simulink model "MX_Gateway"  (Simulink 9.0)
settings: Solver=FixedStepDiscrete  StartTime=0.0  StopTime=100  FixedStep=0.01  RelTol=1e-3
values from neighbouring files (symbols this model uses):
  ACTIVE = Bobcat.Parameter; CreateDefine( ACTIVE                         ,1                          ,'Define' ,'defines.h' )    [defines.m]
  ACTIVE_MODE_ONE = Bobcat.Parameter; CreateDefine( ACTIVE_MODE_ONE                ,19                         ,'Define' ,'defines.h' )    [defines.m]
  AUTHENTICATED = Bobcat.Parameter; CreateDefine( AUTHENTICATED                  ,1                          ,'Define' ,'defines.h' )    [defines.m]
  AUX_EMEA_CONTROLS = Bobcat.Parameter; CreateDefine( AUX_EMEA_CONTROLS  ,1   ,'Define' ,'defines.h' )    [enums.m]
  AUX_FLOW_HIGH = Bobcat.Parameter; CreateDefine( AUX_FLOW_HIGH           ,4  ,'Define' ,'defines.h' )    [enums.m]
  AUX_FLOW_INACTIVE = Bobcat.Parameter; CreateDefine( AUX_FLOW_INACTIVE       ,0  ,'Define' ,'defines.h' )    [enums.m]
  AUX_FLOW_LOW = Bobcat.Parameter; CreateDefine( AUX_FLOW_LOW            ,1  ,'Define' ,'defines.h' )    [enums.m]
  AUX_FLOW_MEDIUM = Bobcat.Parameter; CreateDefine( AUX_FLOW_MEDIUM         ,2  ,'Define' ,'defines.h' )    [enums.m]
  AUX_FLOW_STANDARD = Bobcat.Parameter; CreateDefine( AUX_FLOW_STANDARD       ,3  ,'Define' ,'defines.h' )    [enums.m]
  AUX_NON_FUNCTIONAL = Bobcat.Parameter; CreateDefine( AUX_NON_FUNCTIONAL ,255 ,'Define' ,'defines.h' )    [enums.m]
  BASE_ACTIVE = Bobcat.Parameter; CreateDefine( BASE_ACTIVE                    ,253                        ,'Define' ,'defines.h' )    [defines.m]
  BASE_DETENT = Bobcat.Parameter; CreateDefine( BASE_DETENT                    ,1                          ,'Define' ,'defines.h' )    [defines.m]
  BEEP_NONE = Bobcat.Parameter; CreateDefine( BEEP_NONE                      ,255                        ,'Define' ,'defines.h' )    [defines.m]
  CD_AIR_FILTER_DEBOUNCE_NORMAL = Bobcat.Parameter; CreateAliasCD( CD_AIR_FILTER_DEBOUNCE_NORMAL                        ,uint16(500)                ,'ConstantDataPointer' ,'CONSTANT_DATA' ,'AIR_FILTER_DEBOUNCE_NORMAL')    [cdata.m]    (also in hc12_cdata.m; m4_cdata.m)
  CD_AIR_FILTER_DEBOUNCE_NOT_CONNECTED = Bobcat.Parameter; CreateAliasCD( CD_AIR_FILTER_DEBOUNCE_NOT_CONNECTED                 ,uint16(3000)               ,'ConstantDataPointer' ,'CONSTANT_DATA' ,'AIR_FILTER_DEBOUNCE_NOT_CONNECTED')    [cdata.m]    (also in hc12_cdata.m; m4_cdata.m)
  CD_AIR_FILTER_DEBOUNCE_PLUGGED = Bobcat.Parameter; CreateAliasCD( CD_AIR_FILTER_DEBOUNCE_PLUGGED                       ,uint16(3000)               ,'ConstantDataPointer' ,'CONSTANT_DATA' ,'AIR_FILTER_DEBOUNCE_PLUGGED')    [cdata.m]    (also in hc12_cdata.m; m4_cdata.m)
  CD_AIR_FILTER_FUNCTION = Bobcat.Parameter; CreateAliasCD( CD_AIR_FILTER_FUNCTION                               ,uint8(255)                 ,'ConstantDataPointer' ,'CONSTANT_DATA' ,'AIR_FILTER_FUNCTION')    [cdata.m]    (also in hc12_cdata.m; m4_cdata.m)
  CD_AIR_FILTER_PLUGGED_WEIGHT = Bobcat.Parameter; CreateAliasCD( CD_AIR_FILTER_PLUGGED_WEIGHT                         ,uint8(2)                   ,'ConstantDataPointer' ,'CONSTANT_DATA' ,'AIR_FILTER_PLUGGED_WEIGHT')    [cdata.m]    (also in hc12_cdata.m; m4_cdata.m)
  CD_AIR_FILTER_UNPLUGGED_WEIGHT = Bobcat.Parameter; CreateAliasCD( CD_AIR_FILTER_UNPLUGGED_WEIGHT                       ,uint8(1)                   ,'ConstantDataPointer' ,'CONSTANT_DATA' ,'AIR_FILTER_UNPLUGGED_WEIGHT')    [cdata.m]    (also in hc12_cdata.m; m4_cdata.m)
  CD_AUTOIDLE_ARMBAR_DELAY = Bobcat.Parameter; CreateAliasCD( CD_AUTOIDLE_ARMBAR_DELAY                             ,uint8(2)                   ,'ConstantDataPointer' ,'CONSTANT_DATA' ,'AUTOIDLE_ARMBAR_DELAY')    [cdata.m]    (also in hc12_cdata.m; m4_cdata.m)
  CD_AUTOIDLE_FUNCTION = Bobcat.Parameter; CreateAliasCD( CD_AUTOIDLE_FUNCTION                                 ,uint8(255)                 ,'ConstantDataPointer' ,'CONSTANT_DATA' ,'AUTOIDLE_FUNCTION')    [cdata.m]    (also in hc12_cdata.m; m4_cdata.m)
  CD_AUTOIDLE_IDLE_DELAY = Bobcat.Parameter; CreateAliasCD( CD_AUTOIDLE_IDLE_DELAY                               ,uint8(40)                  ,'ConstantDataPointer' ,'CONSTANT_DATA' ,'AUTOIDLE_IDLE_DELAY')    [cdata.m]    (also in hc12_cdata.m; m4_cdata.m)
  CD_AUTOIDLE_LOW_IDLE_START = Bobcat.Parameter; CreateAliasCD( CD_AUTOIDLE_LOW_IDLE_START                           ,uint8(255)                 ,'ConstantDataPointer' ,'CONSTANT_DATA' ,'AUTOIDLE_LOW_IDLE_START')    [cdata.m]    (also in hc12_cdata.m; m4_cdata.m)
  CD_AUTOIDLE_PRESSURE_OFF = Bobcat.Parameter; CreateAliasCD( CD_AUTOIDLE_PRESSURE_OFF                             ,uint16(300)                ,'ConstantDataPointer' ,'CONSTANT_DATA' ,'AUTOIDLE_PRESSURE_OFF')    [cdata.m]    (also in hc12_cdata.m; m4_cdata.m)
  CD_AUTOIDLE_PRESSURE_ON = Bobcat.Parameter; CreateAliasCD( CD_AUTOIDLE_PRESSURE_ON                              ,uint16(270)                ,'ConstantDataPointer' ,'CONSTANT_DATA' ,'AUTOIDLE_PRESSURE_ON')    [cdata.m]    (also in hc12_cdata.m; m4_cdata.m)
  CD_AUTOSHIFT_FUNCTION = Bobcat.Parameter; CreateAliasCD( CD_AUTOSHIFT_FUNCTION                                ,uint8(255)                 ,'ConstantDataPointer' ,'CONSTANT_DATA' ,'AUTOSHIFT_FUNCTION')    [cdata.m]    (also in hc12_cdata.m; m4_cdata.m)
  CD_AUTOSHIFT_PHIGH1 = Bobcat.Parameter; CreateAliasCD( CD_AUTOSHIFT_PHIGH1                                  ,uint16(205)                ,'ConstantDataPointer' ,'CONSTANT_DATA' ,'AUTOSHIFT_PHIGH1')    [cdata.m]    (also in hc12_cdata.m; m4_cdata.m)
  CD_AUTOSHIFT_PHIGH2 = Bobcat.Parameter; CreateAliasCD( CD_AUTOSHIFT_PHIGH2                                  ,uint16(193)                ,'ConstantDataPointer' ,'CONSTANT_DATA' ,'AUTOSHIFT_PHIGH2')    [cdata.m]    (also in hc12_cdata.m; m4_cdata.m)
  CD_AUTOSHIFT_PHIGH_TEMP1 = Bobcat.Parameter; CreateAliasCD( CD_AUTOSHIFT_PHIGH_TEMP1                             ,int16(70)                  ,'ConstantDataPointer' ,'CONSTANT_DATA' ,'AUTOSHIFT_PHIGH_TEMP1')    [cdata.m]    (also in hc12_cdata.m; m4_cdata.m)
  CD_AUTOSHIFT_PHIGH_TEMP2 = Bobcat.Parameter; CreateAliasCD( CD_AUTOSHIFT_PHIGH_TEMP2                             ,int16(160)                 ,'ConstantDataPointer' ,'CONSTANT_DATA' ,'AUTOSHIFT_PHIGH_TEMP2')    [cdata.m]    (also in hc12_cdata.m; m4_cdata.m)
  CD_AUTOSHIFT_PLOW1 = Bobcat.Parameter; CreateAliasCD( CD_AUTOSHIFT_PLOW1                                   ,uint16(130)                ,'ConstantDataPointer' ,'CONSTANT_DATA' ,'AUTOSHIFT_PLOW1')    [cdata.m]    (also in hc12_cdata.m; m4_cdata.m)
  CD_AUTOSHIFT_PLOW2 = Bobcat.Parameter; CreateAliasCD( CD_AUTOSHIFT_PLOW2                                   ,uint16(100)                ,'ConstantDataPointer' ,'CONSTANT_DATA' ,'AUTOSHIFT_PLOW2')    [cdata.m]    (also in hc12_cdata.m; m4_cdata.m)
  CD_AUTOSHIFT_PLOW_TEMP1 = Bobcat.Parameter; CreateAliasCD( CD_AUTOSHIFT_PLOW_TEMP1                              ,int16(20)                  ,'ConstantDataPointer' ,'CONSTANT_DATA' ,'AUTOSHIFT_PLOW_TEMP1')    [cdata.m]    (also in hc12_cdata.m; m4_cdata.m)
  CD_AUTOSHIFT_PLOW_TEMP2 = Bobcat.Parameter; CreateAliasCD( CD_AUTOSHIFT_PLOW_TEMP2                              ,int16(80)                  ,'ConstantDataPointer' ,'CONSTANT_DATA' ,'AUTOSHIFT_PLOW_TEMP2')    [cdata.m]    (also in hc12_cdata.m; m4_cdata.m)
  CD_AUTOSHIFT_TRAVEL_DELAY = Bobcat.Parameter; CreateAliasCD( CD_AUTOSHIFT_TRAVEL_DELAY                            ,uint8(32)                  ,'ConstantDataPointer' ,'CONSTANT_DATA' ,'AUTOSHIFT_TRAVEL_DELAY')    [cdata.m]    (also in hc12_cdata.m; m4_cdata.m)
  CD_AUX2_CRACKPOINT_CURRENT = Bobcat.Parameter; CreateAliasCD( CD_AUX2_CRACKPOINT_CURRENT                           ,fi(0,0,16,12,'hex','0c28') ,'ConstantDataPointer' ,'CONSTANT_DATA' ,'AUX2_CRACKPOINT_CURRENT')    [cdata.m]    (also in hc12_cdata.m; m4_cdata.m)
  CD_AUX2_FLOW_LOW_FUNCTION = Bobcat.Parameter; CreateAliasCD( CD_AUX2_FLOW_LOW_FUNCTION                            ,uint8(255)                 ,'ConstantDataPointer' ,'CONSTANT_DATA' ,'AUX2_FLOW_LOW_FUNCTION')    [cdata.m]    (also in hc12_cdata.m; m4_cdata.m)
  CD_AUX2_FLOW_MED_FUNCTION = Bobcat.Parameter; CreateAliasCD( CD_AUX2_FLOW_MED_FUNCTION                            ,uint8(255)                 ,'ConstantDataPointer' ,'CONSTANT_DATA' ,'AUX2_FLOW_MED_FUNCTION')    [cdata.m]    (also in hc12_cdata.m; m4_cdata.m)
  CD_AUX2_HI_FLOW_PERCENT = Bobcat.Parameter; CreateAliasCD( CD_AUX2_HI_FLOW_PERCENT                              ,fi(0,0,16,16,'hex','ffff') ,'ConstantDataPointer' ,'CONSTANT_DATA' ,'AUX2_HI_FLOW_PERCENT')    [cdata.m]    (also in hc12_cdata.m; m4_cdata.m)
  CD_AUX2_LOW_FLOW_PERCENT = Bobcat.Parameter; CreateAliasCD( CD_AUX2_LOW_FLOW_PERCENT                             ,fi(0,0,16,16,'hex','7333') ,'ConstantDataPointer' ,'CONSTANT_DATA' ,'AUX2_LOW_FLOW_PERCENT')    [cdata.m]    (also in hc12_cdata.m; m4_cdata.m)
  CD_AUX2_MAX_FLOW_CURRENT = Bobcat.Parameter; CreateAliasCD( CD_AUX2_MAX_FLOW_CURRENT                             ,fi(0,0,16,12,'hex','14cc') ,'ConstantDataPointer' ,'CONSTANT_DATA' ,'AUX2_MAX_FLOW_CURRENT')    [cdata.m]    (also in hc12_cdata.m; m4_cdata.m)
  CD_AUX2_MED_FLOW_PERCENT = Bobcat.Parameter; CreateAliasCD( CD_AUX2_MED_FLOW_PERCENT                             ,fi(0,0,16,16,'hex','9999') ,'ConstantDataPointer' ,'CONSTANT_DATA' ,'AUX2_MED_FLOW_PERCENT')    [cdata.m]    (also in hc12_cdata.m; m4_cdata.m)
  CD_AUX_CRACKPOINT_CURRENT = Bobcat.Parameter; CreateAliasCD( CD_AUX_CRACKPOINT_CURRENT                            ,fi(0,0,16,12,'hex','0000') ,'ConstantDataPointer' ,'CONSTANT_DATA' ,'AUX_CRACKPOINT_CURRENT')    [cdata.m]    (also in hc12_cdata.m; m4_cdata.m)
  CD_AUX_DETENT_FLASH_TIME = Bobcat.Parameter; CreateAliasCD( CD_AUX_DETENT_FLASH_TIME                             ,uint16(72)                 ,'ConstantDataPointer' ,'CONSTANT_DATA' ,'AUX_DETENT_FLASH_TIME')    [cdata.m]    (also in hc12_cdata.m; m4_cdata.m)
  CD_AUX_DETENT_HOLD_TIME = Bobcat.Parameter; CreateAliasCD( CD_AUX_DETENT_HOLD_TIME                              ,uint16(100)                ,'ConstantDataPointer' ,'CONSTANT_DATA' ,'AUX_DETENT_HOLD_TIME')    [cdata.m]    (also in hc12_cdata.m; m4_cdata.m)
  CD_AUX_FULL_ON_AUX1 = Bobcat.Parameter; CreateAliasCD( CD_AUX_FULL_ON_AUX1                                  ,fi(0,0,16,12,'hex','10cd') ,'ConstantDataPointer' ,'CONSTANT_DATA' ,'AUX_FULL_ON_AUX1')    [cdata.m]    (also in hc12_cdata.m; m4_cdata.m)
  CD_AUX_FULL_ON_AUX2 = Bobcat.Parameter; CreateAliasCD( CD_AUX_FULL_ON_AUX2                                  ,fi(0,0,16,12,'hex','139d') ,'ConstantDataPointer' ,'CONSTANT_DATA' ,'AUX_FULL_ON_AUX2')    [cdata.m]    (also in hc12_cdata.m; m4_cdata.m)
  CD_AUX_FULL_ON_AUX3 = Bobcat.Parameter; CreateAliasCD( CD_AUX_FULL_ON_AUX3                                  ,fi(0,0,16,12,'hex','17d7') ,'ConstantDataPointer' ,'CONSTANT_DATA' ,'AUX_FULL_ON_AUX3')    [cdata.m]    (also in hc12_cdata.m; m4_cdata.m)
  CD_AUX_FUNCTION = Bobcat.Parameter; CreateAliasCD( CD_AUX_FUNCTION                                      ,uint8(0)                   ,'ConstantDataPointer' ,'CONSTANT_DATA' ,'AUX_FUNCTION')    [cdata.m]    (also in hc12_cdata.m; m4_cdata.m)
  CD_AUX_RATE_LIMIT_CRACKPOINT = Bobcat.Parameter; CreateAliasCD( CD_AUX_RATE_LIMIT_CRACKPOINT                         ,fi(0,0,16,12,'hex','0800') ,'ConstantDataPointer' ,'CONSTANT_DATA' ,'AUX_RATE_LIMIT_CRACKPOINT')    [cdata.m]    (also in hc12_cdata.m; m4_cdata.m)
  CD_AUX_RATE_LIMIT_CRACKPOINT_RATE = Bobcat.Parameter; CreateAliasCD( CD_AUX_RATE_LIMIT_CRACKPOINT_RATE                    ,fi(0,1,32,12,'int',102400) ,'ConstantDataPointer' ,'CONSTANT_DATA' ,'AUX_RATE_LIMIT_CRACKPOINT_RATE')    [cdata.m]    (also in hc12_cdata.m; m4_cdata.m)
  CD_AUX_RATE_LIMIT_FUNCTION = Bobcat.Parameter; CreateAliasCD( CD_AUX_RATE_LIMIT_FUNCTION                           ,uint8(255)                 ,'ConstantDataPointer' ,'CONSTANT_DATA' ,'AUX_RATE_LIMIT_FUNCTION')    [cdata.m]    (also in hc12_cdata.m; m4_cdata.m)
  CD_AUX_RATE_LIMIT_MAX_ENG_SPD = Bobcat.Parameter; CreateAliasCD( CD_AUX_RATE_LIMIT_MAX_ENG_SPD                        ,uint16(1600)               ,'ConstantDataPointer' ,'CONSTANT_DATA' ,'AUX_RATE_LIMIT_MAX_ENG_SPD')    [cdata.m]    (also in hc12_cdata.m; m4_cdata.m)
  CD_AUX_RATE_LIMIT_MIN_ENG_SPD = Bobcat.Parameter; CreateAliasCD( CD_AUX_RATE_LIMIT_MIN_ENG_SPD                        ,uint16(100)                ,'ConstantDataPointer' ,'CONSTANT_DATA' ,'AUX_RATE_LIMIT_MIN_ENG_SPD')    [cdata.m]    (also in hc12_cdata.m; m4_cdata.m)
  CD_AUX_RATE_LIMIT_NORMAL_RATE = Bobcat.Parameter; CreateAliasCD( CD_AUX_RATE_LIMIT_NORMAL_RATE                        ,fi(0,1,32,12,'int',2048)   ,'ConstantDataPointer' ,'CONSTANT_DATA' ,'AUX_RATE_LIMIT_NORMAL_RATE')    [cdata.m]    (also in hc12_cdata.m; m4_cdata.m)
  CD_AUXPOT_CAL_DEADBAND_HIGH = Bobcat.Parameter; CreateAliasCD( CD_AUXPOT_CAL_DEADBAND_HIGH                          ,uint16(574)                ,'ConstantDataPointer' ,'CONSTANT_DATA' ,'AUXPOT_CAL_DEADBAND_HIGH')    [cdata.m]    (also in hc12_cdata.m; m4_cdata.m)
  CD_AUXPOT_CAL_DEADBAND_LOW = Bobcat.Parameter; CreateAliasCD( CD_AUXPOT_CAL_DEADBAND_LOW                           ,uint16(424)                ,'ConstantDataPointer' ,'CONSTANT_DATA' ,'AUXPOT_CAL_DEADBAND_LOW')    [cdata.m]    (also in hc12_cdata.m; m4_cdata.m)
  CD_AUXPOT_CENTER_RATIO = Bobcat.Parameter; CreateAliasCD( CD_AUXPOT_CENTER_RATIO                               ,uint16(500)                ,'ConstantDataPointer' ,'CONSTANT_DATA' ,'AUXPOT_CENTER_RATIO')    [cdata.m]    (also in hc12_cdata.m; m4_cdata.m)
  CD_AUXPOT_DEADBAND = Bobcat.Parameter; CreateAliasCD( CD_AUXPOT_DEADBAND                                   ,uint8(38)                  ,'ConstantDataPointer' ,'CONSTANT_DATA' ,'AUXPOT_DEADBAND')    [cdata.m]    (also in hc12_cdata.m; m4_cdata.m)
  CD_AUXPOT_FUNCTION = Bobcat.Parameter; CreateAliasCD( CD_AUXPOT_FUNCTION                                   ,uint8(0)                   ,'ConstantDataPointer' ,'CONSTANT_DATA' ,'AUXPOT_FUNCTION')    [cdata.m]    (also in hc12_cdata.m; m4_cdata.m)
  CD_AUXPOT_RATIO_GAIN = Bobcat.Parameter; CreateAliasCD( CD_AUXPOT_RATIO_GAIN                                 ,uint16(1000)               ,'ConstantDataPointer' ,'CONSTANT_DATA' ,'AUXPOT_RATIO_GAIN')    [cdata.m]    (also in hc12_cdata.m; m4_cdata.m)
  CD_CAN_THROTTLE_FUNCTION = Bobcat.Parameter; CreateAliasCD( CD_CAN_THROTTLE_FUNCTION                             ,uint8(255)                 ,'ConstantDataPointer' ,'CONSTANT_DATA' ,'CAN_THROTTLE_FUNCTION')    [cdata.m]    (also in hc12_cdata.m; m4_cdata.m)
  CD_CAN_THROTTLE_REVERSE = Bobcat.Parameter; CreateAliasCD( CD_CAN_THROTTLE_REVERSE                              ,uint8(255)                 ,'ConstantDataPointer' ,'CONSTANT_DATA' ,'CAN_THROTTLE_REVERSE')    [cdata.m]    (also in hc12_cdata.m; m4_cdata.m)
  CD_CONSOLE_DEBOUNCE_ABSENT = Bobcat.Parameter; CreateAliasCD( CD_CONSOLE_DEBOUNCE_ABSENT                           ,uint16(12)                 ,'ConstantDataPointer' ,'CONSTANT_DATA' ,'CONSOLE_DEBOUNCE_ABSENT')    [cdata.m]    (also in hc12_cdata.m; m4_cdata.m)
  CD_CONSOLE_DEBOUNCE_OORH = Bobcat.Parameter; CreateAliasCD( CD_CONSOLE_DEBOUNCE_OORH                             ,uint16(12)                 ,'ConstantDataPointer' ,'CONSTANT_DATA' ,'CONSOLE_DEBOUNCE_OORH')    [cdata.m]    (also in hc12_cdata.m; m4_cdata.m)
  CD_CONSOLE_DEBOUNCE_OORL = Bobcat.Parameter; CreateAliasCD( CD_CONSOLE_DEBOUNCE_OORL                             ,uint16(12)                 ,'ConstantDataPointer' ,'CONSTANT_DATA' ,'CONSOLE_DEBOUNCE_OORL')    [cdata.m]    (also in hc12_cdata.m; m4_cdata.m)
  CD_CONSOLE_DEBOUNCE_PRESENT = Bobcat.Parameter; CreateAliasCD( CD_CONSOLE_DEBOUNCE_PRESENT                          ,uint16(12)                 ,'ConstantDataPointer' ,'CONSTANT_DATA' ,'CONSOLE_DEBOUNCE_PRESENT')    [cdata.m]    (also in hc12_cdata.m; m4_cdata.m)
  CD_CONSOLE_FUNCTION = Bobcat.Parameter; CreateAliasCD( CD_CONSOLE_FUNCTION                                  ,uint8(0)                   ,'ConstantDataPointer' ,'CONSTANT_DATA' ,'CONSOLE_FUNCTION')    [cdata.m]    (also in hc12_cdata.m; m4_cdata.m)
  CD_CONSOLE_OORH = Bobcat.Parameter; CreateAliasCD( CD_CONSOLE_OORH                                      ,uint16(740)                ,'ConstantDataPointer' ,'CONSTANT_DATA' ,'CONSOLE_OORH')    [cdata.m]    (also in hc12_cdata.m; m4_cdata.m)
  CD_CONSOLE_OORL = Bobcat.Parameter; CreateAliasCD( CD_CONSOLE_OORL                                      ,uint16(10)                 ,'ConstantDataPointer' ,'CONSTANT_DATA' ,'CONSOLE_OORL')    [cdata.m]    (also in hc12_cdata.m; m4_cdata.m)
  CD_CONSOLE_PRESENT_HIGH = Bobcat.Parameter; CreateAliasCD( CD_CONSOLE_PRESENT_HIGH                              ,uint16(515)                ,'ConstantDataPointer' ,'CONSTANT_DATA' ,'CONSOLE_PRESENT_HIGH')    [cdata.m]    (also in hc12_cdata.m; m4_cdata.m)
  CD_CONSOLE_PRESENT_LOW = Bobcat.Parameter; CreateAliasCD( CD_CONSOLE_PRESENT_LOW                               ,uint16(375)                ,'ConstantDataPointer' ,'CONSTANT_DATA' ,'CONSOLE_PRESENT_LOW')    [cdata.m]    (also in hc12_cdata.m; m4_cdata.m)
  CD_DELUXE_GEN5_5_FUNCTION = Bobcat.Parameter; CreateAliasCD( CD_DELUXE_GEN5_5_FUNCTION                            ,uint8(255)                 ,'ConstantDataPointer' ,'CONSTANT_DATA' ,'DELUXE_GEN5_5_FUNCTION')    [cdata.m]    (also in hc12_cdata.m; m4_cdata.m)
  CD_DELUXE_GEN5_FUNCTION = Bobcat.Parameter; CreateAliasCD( CD_DELUXE_GEN5_FUNCTION                              ,uint8(255)                 ,'ConstantDataPointer' ,'CONSTANT_DATA' ,'DELUXE_GEN5_FUNCTION')    [cdata.m]    (also in hc12_cdata.m; m4_cdata.m)
  CD_DERATE_ENGINE_SPEED_DEBOUNCE_HIGH = Bobcat.Parameter; CreateAliasCD( CD_DERATE_ENGINE_SPEED_DEBOUNCE_HIGH                 ,uint16(200)                ,'ConstantDataPointer' ,'CONSTANT_DATA' ,'DERATE_ENGINE_SPEED_DEBOUNCE_HIGH')    [cdata.m]    (also in hc12_cdata.m; m4_cdata.m)
  CD_DERATE_ENGINE_SPEED_DEBOUNCE_SHUTDOWN = Bobcat.Parameter; CreateAliasCD( CD_DERATE_ENGINE_SPEED_DEBOUNCE_SHUTDOWN             ,uint16(1000)               ,'ConstantDataPointer' ,'CONSTANT_DATA' ,'DERATE_ENGINE_SPEED_DEBOUNCE_SHUTDOWN')    [cdata.m]    (also in hc12_cdata.m; m4_cdata.m)
  CD_DERATE_ENGINE_SPEED_HIGH_OFFSET = Bobcat.Parameter; CreateAliasCD( CD_DERATE_ENGINE_SPEED_HIGH_OFFSET                   ,uint16(200)                ,'ConstantDataPointer' ,'CONSTANT_DATA' ,'DERATE_ENGINE_SPEED_HIGH_OFFSET')    [cdata.m]    (also in hc12_cdata.m; m4_cdata.m)
  CD_DERATE_METHOD = Bobcat.Parameter; CreateAliasCD( CD_DERATE_METHOD                                     ,uint8(0)                   ,'ConstantDataPointer' ,'CONSTANT_DATA' ,'DERATE_METHOD')    [cdata.m]    (also in hc12_cdata.m; m4_cdata.m)
  CD_DUMP_VALVE_FUNCTION = Bobcat.Parameter; CreateAliasCD( CD_DUMP_VALVE_FUNCTION                               ,uint8(255)                 ,'ConstantDataPointer' ,'CONSTANT_DATA' ,'DUMP_VALVE_FUNCTION')    [cdata.m]    (also in hc12_cdata.m; m4_cdata.m)
  CD_DUMP_VALVE_ON_CURRENT = Bobcat.Parameter; CreateAliasCD( CD_DUMP_VALVE_ON_CURRENT                             ,fi(0,0,16,12,'hex','ffff') ,'ConstantDataPointer' ,'CONSTANT_DATA' ,'DUMP_VALVE_ON_CURRENT')    [cdata.m]    (also in hc12_cdata.m; m4_cdata.m)
  CD_ECO_MODE_THROTTLE_REDUCTION = Bobcat.Parameter; CreateAliasCD( CD_ECO_MODE_THROTTLE_REDUCTION                       ,uint16(850)                ,'ConstantDataPointer' ,'CONSTANT_DATA' ,'ECO_MODE_THROTTLE_REDUCTION')    [cdata.m]    (also in hc12_cdata.m; m4_cdata.m)
  CD_ECT_FILTER_GAIN = Bobcat.Parameter; CreateAliasCD( CD_ECT_FILTER_GAIN                                   ,uint8(6)                   ,'ConstantDataPointer' ,'CONSTANT_DATA' ,'ECT_FILTER_GAIN')    [cdata.m]    (also in hc12_cdata.m; m4_cdata.m)
  CD_ECU_FUNCTION = Bobcat.Parameter; CreateAliasCD( CD_ECU_FUNCTION                                      ,uint8(255)                 ,'ConstantDataPointer' ,'CONSTANT_DATA' ,'ECU_FUNCTION')    [cdata.m]    (also in hc12_cdata.m; m4_cdata.m)
  CD_ECU_HOLD_DEBOUNCE_ERROR_ON = Bobcat.Parameter; CreateAliasCD( CD_ECU_HOLD_DEBOUNCE_ERROR_ON                        ,uint16(20)                 ,'ConstantDataPointer' ,'CONSTANT_DATA' ,'ECU_HOLD_DEBOUNCE_ERROR_ON')    [cdata.m]    (also in hc12_cdata.m; m4_cdata.m)
  CD_EMEA_CONTROLS_HOLD_TIME = Bobcat.Parameter; CreateAliasCD( CD_EMEA_CONTROLS_HOLD_TIME                           ,uint16(30)                 ,'ConstantDataPointer' ,'CONSTANT_DATA' ,'EMEA_CONTROLS_HOLD_TIME')    [cdata.m]    (also in hc12_cdata.m; m4_cdata.m)
  CD_EMEA_CONTROLS_OPTION_A = Bobcat.Parameter; CreateAliasCD( CD_EMEA_CONTROLS_OPTION_A                            ,uint8(255)                 ,'ConstantDataPointer' ,'CONSTANT_DATA' ,'EMEA_CONTROLS_OPTION_A')    [cdata.m]    (also in hc12_cdata.m; m4_cdata.m)
  CD_EMEA_CONTROLS_OPTION_C = Bobcat.Parameter; CreateAliasCD( CD_EMEA_CONTROLS_OPTION_C                            ,uint8(255)                 ,'ConstantDataPointer' ,'CONSTANT_DATA' ,'EMEA_CONTROLS_OPTION_C')    [cdata.m]    (also in hc12_cdata.m; m4_cdata.m)
  CD_EMEA_CONTROLS_REVERSE = Bobcat.Parameter; CreateAliasCD( CD_EMEA_CONTROLS_REVERSE                             ,uint8(255)                 ,'ConstantDataPointer' ,'CONSTANT_DATA' ,'EMEA_CONTROLS_REVERSE')    [cdata.m]    (also in hc12_cdata.m; m4_cdata.m)
  CD_EMEA_ELECTRIC_VERSION = Bobcat.Parameter; CreateAliasCD( CD_EMEA_ELECTRIC_VERSION                             ,uint8(0)                   ,'ConstantDataPointer' ,'CONSTANT_DATA' ,'EMEA_ELECTRIC_VERSION')    [cdata.m]    (also in hc12_cdata.m; m4_cdata.m)
  CD_EMEA_POT_CAL_DEADBAND_HIGH = Bobcat.Parameter; CreateAliasCD( CD_EMEA_POT_CAL_DEADBAND_HIGH                        ,uint16(574)                ,'ConstantDataPointer' ,'CONSTANT_DATA' ,'EMEA_POT_CAL_DEADBAND_HIGH')    [cdata.m]    (also in hc12_cdata.m; m4_cdata.m)
  CD_EMEA_POT_CAL_DEADBAND_LOW = Bobcat.Parameter; CreateAliasCD( CD_EMEA_POT_CAL_DEADBAND_LOW                         ,uint16(424)                ,'ConstantDataPointer' ,'CONSTANT_DATA' ,'EMEA_POT_CAL_DEADBAND_LOW')    [cdata.m]    (also in hc12_cdata.m; m4_cdata.m)
  CD_ENGINE_RPM_FACTOR = Bobcat.Parameter; CreateAliasCD( CD_ENGINE_RPM_FACTOR                                 ,uint8(35)                  ,'ConstantDataPointer' ,'CONSTANT_DATA' ,'ENGINE_RPM_FACTOR')    [cdata.m]    (also in hc12_cdata.m; m4_cdata.m)
  CD_ENGINE_SPEED_FILTER_FAST_VALUE = Bobcat.Parameter; CreateAliasCD( CD_ENGINE_SPEED_FILTER_FAST_VALUE                    ,uint8(1)                   ,'ConstantDataPointer' ,'CONSTANT_DATA' ,'ENGINE_SPEED_FILTER_FAST_VALUE')    [cdata.m]    (also in hc12_cdata.m; m4_cdata.m)
  CD_ENGINE_SPEED_FILTER_SLOW_VALUE = Bobcat.Parameter; CreateAliasCD( CD_ENGINE_SPEED_FILTER_SLOW_VALUE                    ,uint8(4)                   ,'ConstantDataPointer' ,'CONSTANT_DATA' ,'ENGINE_SPEED_FILTER_SLOW_VALUE')    [cdata.m]    (also in hc12_cdata.m; m4_cdata.m)
  CD_ENGINE_SPEED_RUNNING = Bobcat.Parameter; CreateAliasCD( CD_ENGINE_SPEED_RUNNING                              ,uint16(800)                ,'ConstantDataPointer' ,'CONSTANT_DATA' ,'ENGINE_SPEED_RUNNING')    [cdata.m]    (also in hc12_cdata.m; m4_cdata.m)
  CD_ENGINE_START_DELAY_DEBOUNCE_RPM_NO_SIGNAL = Bobcat.Parameter; CreateAliasCD( CD_ENGINE_START_DELAY_DEBOUNCE_RPM_NO_SIGNAL         ,uint16(0)                  ,'ConstantDataPointer' ,'CONSTANT_DATA' ,'ENGINE_START_DELAY_DEBOUNCE_RPM_NO_SIGNAL')    [cdata.m]    (also in hc12_cdata.m; m4_cdata.m)
  CD_ENGINE_START_DELAY_OFF_TIME = Bobcat.Parameter; CreateAliasCD( CD_ENGINE_START_DELAY_OFF_TIME                       ,uint16(0)                  ,'ConstantDataPointer' ,'CONSTANT_DATA' ,'ENGINE_START_DELAY_OFF_TIME')    [cdata.m]    (also in hc12_cdata.m; m4_cdata.m)
  CD_ENGINE_START_DELAY_RPM_NO_SIGNAL = Bobcat.Parameter; CreateAliasCD( CD_ENGINE_START_DELAY_RPM_NO_SIGNAL                  ,uint16(0)                  ,'ConstantDataPointer' ,'CONSTANT_DATA' ,'ENGINE_START_DELAY_RPM_NO_SIGNAL')    [cdata.m]    (also in hc12_cdata.m; m4_cdata.m)
  CD_EOP_DEBOUNCE_EXTREMELY_LOW = Bobcat.Parameter; CreateAliasCD( CD_EOP_DEBOUNCE_EXTREMELY_LOW                        ,uint16(50)                 ,'ConstantDataPointer' ,'CONSTANT_DATA' ,'EOP_DEBOUNCE_EXTREMELY_LOW')    [cdata.m]    (also in hc12_cdata.m; m4_cdata.m)
  CD_EOP_DEBOUNCE_NORMAL = Bobcat.Parameter; CreateAliasCD( CD_EOP_DEBOUNCE_NORMAL                               ,uint16(50)                 ,'ConstantDataPointer' ,'CONSTANT_DATA' ,'EOP_DEBOUNCE_NORMAL')    [cdata.m]    (also in hc12_cdata.m; m4_cdata.m)
  CD_EOP_DEBOUNCE_SHUTDOWN = Bobcat.Parameter; CreateAliasCD( CD_EOP_DEBOUNCE_SHUTDOWN                             ,uint16(100)                ,'ConstantDataPointer' ,'CONSTANT_DATA' ,'EOP_DEBOUNCE_SHUTDOWN')    [cdata.m]    (also in hc12_cdata.m; m4_cdata.m)
  CD_EOP_FILTER_VALUE = Bobcat.Parameter; CreateAliasCD( CD_EOP_FILTER_VALUE                                  ,uint8(3)                   ,'ConstantDataPointer' ,'CONSTANT_DATA' ,'EOP_FILTER_VALUE')    [cdata.m]    (also in hc12_cdata.m; m4_cdata.m)
  CD_EOP_FUNCTION = Bobcat.Parameter; CreateAliasCD( CD_EOP_FUNCTION                                      ,uint8(1)                   ,'ConstantDataPointer' ,'CONSTANT_DATA' ,'EOP_FUNCTION')    [cdata.m]    (also in hc12_cdata.m; m4_cdata.m)
  CD_EOP_SWITCH_GAUGE_VALUE = Bobcat.Parameter; CreateAliasCD( CD_EOP_SWITCH_GAUGE_VALUE                            ,uint16(125)                ,'ConstantDataPointer' ,'CONSTANT_DATA' ,'EOP_SWITCH_GAUGE_VALUE')    [cdata.m]    (also in hc12_cdata.m; m4_cdata.m)
  CD_EOP_SWITCH_LOW = Bobcat.Parameter; CreateAliasCD( CD_EOP_SWITCH_LOW                                    ,uint16(50)                 ,'ConstantDataPointer' ,'CONSTANT_DATA' ,'EOP_SWITCH_LOW')    [cdata.m]    (also in hc12_cdata.m; m4_cdata.m)
  CD_EOP_SWITCH_PRESSURE_VALUE = Bobcat.Parameter; CreateAliasCD( CD_EOP_SWITCH_PRESSURE_VALUE                         ,uint16(40)                 ,'ConstantDataPointer' ,'CONSTANT_DATA' ,'EOP_SWITCH_PRESSURE_VALUE')    [cdata.m]    (also in hc12_cdata.m; m4_cdata.m)
  CD_EOT_DEBOUNCE_EXTREMELY_LOW = Bobcat.Parameter; CreateAliasCD( CD_EOT_DEBOUNCE_EXTREMELY_LOW                        ,uint16(2)                  ,'ConstantDataPointer' ,'CONSTANT_DATA' ,'EOT_DEBOUNCE_EXTREMELY_LOW')    [cdata.m]    (also in hc12_cdata.m; m4_cdata.m)
  CD_EOT_DEBOUNCE_LOW = Bobcat.Parameter; CreateAliasCD( CD_EOT_DEBOUNCE_LOW                                  ,uint16(2)                  ,'ConstantDataPointer' ,'CONSTANT_DATA' ,'EOT_DEBOUNCE_LOW')    [cdata.m]    (also in hc12_cdata.m; m4_cdata.m)
  CD_EOT_DEBOUNCE_NORMAL = Bobcat.Parameter; CreateAliasCD( CD_EOT_DEBOUNCE_NORMAL                               ,uint16(2)                  ,'ConstantDataPointer' ,'CONSTANT_DATA' ,'EOT_DEBOUNCE_NORMAL')    [cdata.m]    (also in hc12_cdata.m; m4_cdata.m)
  CD_EOT_EXTREMELY_LOW = Bobcat.Parameter; CreateAliasCD( CD_EOT_EXTREMELY_LOW                                 ,uint16(81)                 ,'ConstantDataPointer' ,'CONSTANT_DATA' ,'EOT_EXTREMELY_LOW')    [cdata.m]    (also in hc12_cdata.m; m4_cdata.m)
  CD_EOT_FUNCTION = Bobcat.Parameter; CreateAliasCD( CD_EOT_FUNCTION                                      ,uint8(255)                 ,'ConstantDataPointer' ,'CONSTANT_DATA' ,'EOT_FUNCTION')    [cdata.m]    (also in hc12_cdata.m; m4_cdata.m)
  CD_EOT_LOW = Bobcat.Parameter; CreateAliasCD( CD_EOT_LOW                                           ,uint16(90)                 ,'ConstantDataPointer' ,'CONSTANT_DATA' ,'EOT_LOW')    [cdata.m]    (also in hc12_cdata.m; m4_cdata.m)
  CD_EOT_TEMPERATURE_HYSTERESIS = Bobcat.Parameter; CreateAliasCD( CD_EOT_TEMPERATURE_HYSTERESIS                        ,uint16(2)                  ,'ConstantDataPointer' ,'CONSTANT_DATA' ,'EOT_TEMPERATURE_HYSTERESIS')    [cdata.m]    (also in hc12_cdata.m; m4_cdata.m)
  CD_FAST_TRAVEL_FUNCTION = Bobcat.Parameter; CreateAliasCD( CD_FAST_TRAVEL_FUNCTION                              ,uint8(255)                 ,'ConstantDataPointer' ,'CONSTANT_DATA' ,'FAST_TRAVEL_FUNCTION')    [cdata.m]    (also in hc12_cdata.m; m4_cdata.m)
  CD_FAST_TRAVEL_THROTTLE_DELAY = Bobcat.Parameter; CreateAliasCD( CD_FAST_TRAVEL_THROTTLE_DELAY                        ,uint16(10)                 ,'ConstantDataPointer' ,'CONSTANT_DATA' ,'FAST_TRAVEL_THROTTLE_DELAY')    [cdata.m]    (also in hc12_cdata.m; m4_cdata.m)
  CD_FAST_TRAVEL_THROTTLE_LIMIT = Bobcat.Parameter; CreateAliasCD( CD_FAST_TRAVEL_THROTTLE_LIMIT                        ,uint16(1000)               ,'ConstantDataPointer' ,'CONSTANT_DATA' ,'FAST_TRAVEL_THROTTLE_LIMIT')    [cdata.m]    (also in hc12_cdata.m; m4_cdata.m)
  CD_FLYWHEEL_TEETH = Bobcat.Parameter; CreateAliasCD( CD_FLYWHEEL_TEETH                                    ,uint8(83)                  ,'ConstantDataPointer' ,'CONSTANT_DATA' ,'FLYWHEEL_TEETH')    [cdata.m]    (also in hc12_cdata.m; m4_cdata.m)
  CD_FUEL_LEVEL_FILTER_FAST_VALUE = Bobcat.Parameter; CreateAliasCD( CD_FUEL_LEVEL_FILTER_FAST_VALUE                      ,uint8(5)                   ,'ConstantDataPointer' ,'CONSTANT_DATA' ,'FUEL_LEVEL_FILTER_FAST_VALUE')    [cdata.m]    (also in hc12_cdata.m; m4_cdata.m)
  CD_FUEL_LEVEL_FILTER_SLOW_VALUE = Bobcat.Parameter; CreateAliasCD( CD_FUEL_LEVEL_FILTER_SLOW_VALUE                      ,uint8(10)                  ,'ConstantDataPointer' ,'CONSTANT_DATA' ,'FUEL_LEVEL_FILTER_SLOW_VALUE')    [cdata.m]    (also in hc12_cdata.m; m4_cdata.m)
  CD_FUEL_PRIMARY_FEEDBACK_FUNCTION = Bobcat.Parameter; CreateAliasCD( CD_FUEL_PRIMARY_FEEDBACK_FUNCTION                    ,uint8(0)                   ,'ConstantDataPointer' ,'CONSTANT_DATA' ,'FUEL_PRIMARY_FEEDBACK_FUNCTION')    [cdata.m]    (also in hc12_cdata.m; m4_cdata.m)
  ... (+246 more)
undefined symbols (not defined in the file or in its folder): sigbuilder_block
undefined symbols (not defined in the file): CD_FUEL_RESOLUTION_TIMER
libraries: simulink x186, MX_Library x107
(part 31 of 48)

== stateflow chart MX_Gtwy_Control/Outputs/FuelSec_ECUHold/Solenoid ==
  state ECU_HOLD
    state NORMAL
      state CHECK_ERROR_ON
        en:duty_FuelSec = INACTIVE;
        en:state_ECUHold = INACTIVE;
        en:debounceCount = 0;
        du:debounceCount++;
      state ACTIVE
        en:duty_FuelSec = FULL_ON;
        en:state_ECUHold = ACTIVE;
      note: Only go active if the starter output is inactive unless
          | we are in a cranking state.  We do not want the machine
          | to start cranking once key is on if the starter is shorted.
      (default) -> CHECK_ERROR_ON
      ECU_HOLD.NORMAL.ACTIVE -> CHECK_ERROR_ON : [(state_StarterOutput != NORMAL)...
          | || (state_StarterRelay != NORMAL)...
          | || (state_Engine == ENGINE_OFF)]
      CHECK_ERROR_ON -> ECU_HOLD.NORMAL.ACTIVE : [(debounceCount >= CD_ECU_HOLD_DEBOUNCE_ERROR_ON)...
          | && (state_StarterOutput == NORMAL)...
          | && (state_StarterRelay == NORMAL)...
          | && ((din_StarterRelay == INACTIVE) || (state_Engine == ENGINE_CRANKING))...
          | && (state_Engine != ENGINE_OFF)]
    state ERROR
      en:duty_FuelSec = INACTIVE;
      en:state_ECUHold = error_FuelSec;
    (default) -> NORMAL
    NORMAL -> ECU_HOLD.ERROR : [(error_FuelSec != NORMAL)]
    ECU_HOLD.ERROR -> NORMAL : [(error_FuelSec == NORMAL)]
  state NON_FUNCTIONAL
    en:duty_FuelSec = INACTIVE;
    en:state_FuelSec = INACTIVE;
    en:state_ECUHold = INACTIVE;
  state FUEL_HOLD
    state ACTIVE
      state INACTIVE
        en:duty_FuelSec = INACTIVE;
        en:state_FuelSec = INACTIVE;
      state ACTIVE
        en:duty_FuelSec = FULL_ON;
        en:state_FuelSec = ACTIVE;
      (default) -> INACTIVE
      FUEL_HOLD.ACTIVE.ACTIVE -> INACTIVE : [(state_Engine == SHUTDOWN)...
          | || (state_Engine == ENGINE_OFF)]
      INACTIVE -> FUEL_HOLD.ACTIVE.ACTIVE : [state_Engine == ENGINE_CRANKING]
    state ERROR
      en:duty_FuelSec = INACTIVE;
      en:state_FuelSec = error_FuelSec;
    (default) -> FUEL_HOLD.ACTIVE
    FUEL_HOLD.ACTIVE -> FUEL_HOLD.ERROR : [(error_FuelSec != NORMAL)]
    FUEL_HOLD.ERROR -> FUEL_HOLD.ACTIVE : [(error_FuelSec == NORMAL)]
  (default) -> NON_FUNCTIONAL
  NON_FUNCTIONAL -> ECU_HOLD [order 1] : [(CD_ECU_FUNCTION != NON_FUNCTIONAL)...
      | && (state_System == SYS_NORMAL)...
      | && (state_Engine != SHUTDOWN)]
  ECU_HOLD -> NON_FUNCTIONAL : [(state_System != SYS_NORMAL)...
      | || (state_Engine == SHUTDOWN)]
  NON_FUNCTIONAL -> FUEL_HOLD [order 2] : [(CD_FUEL_SECONDARY_FUNCTION != NON_FUNCTIONAL)...
      | && (state_System == SYS_NORMAL)]
  FUEL_HOLD -> NON_FUNCTIONAL : [(state_System != SYS_NORMAL)]
  data: state_Engine input uint8; error_FuelSec input uint8; duty_FuelSec output fixdt(0,16,16); CD_FUEL_SECONDARY_FUNCTION parameter uint8; FULL_ON parameter fixdt(0,16,16); NORMAL parameter uint8; INACTIVE parameter uint8; SHUTDOWN parameter uint8; ENGINE_CRANKING parameter uint8; SYS_NORMAL parameter uint8; state_System input uint8; ENGINE_OFF parameter uint8; state_FuelSec output uint8; ACTIVE parameter uint8; NON_FUNCTIONAL parameter uint8; CD_ECU_FUNCTION parameter uint8; CD_ECU_HOLD_DEBOUNCE_ERROR_ON parameter uint16; state_ECUHold output uint8; debounceCount local uint16; state_StarterOutput input uint8; state_StarterRelay input uint8; din_StarterRelay input boolean

== stateflow chart MX_Gtwy_Control/Outputs/GlowPlug/GlowPlug ==
  state ECU
    state Wait
      en:
      count_GlowPlug = 0;
    state Stop
      en:
      count_GlowPlug = 0;
    state Countdown
      en:
      count_GlowPlug = time_GlowPlug;
      du:
      count_GlowPlug--;
    (default) -> Wait
    Stop -> Wait : [state_Engine == ENGINE_NOT_RUNNING...
        | && ecu_GlowPlug == INACTIVE]
    Wait -> Countdown : [ecu_GlowPlug == ACTIVE...
        | && state_ecuECT == NORMAL]
    Countdown -> Stop : [state_Engine == ENGINE_RUNNING...
        | || ecu_GlowPlug == INACTIVE...
        | || count_GlowPlug == 0 ]
  state NON_FUNCTIONAL
    en:
    dout_GlowPlug = INACTIVE;
    count_GlowPlug = 0;
  state NORMAL
    state GLOW_DURING_CRANK
      en:dout_GlowPlug = ACTIVE;
      en:debounceCrank = 0;
      du:debounceCrank++;
      ex:state_CrankTimerExpired = ACTIVE;
      en:glowPlugAfterGlowDone = INACTIVE;
    state WAIT_STARTUP
      en:dout_GlowPlug = 0;
      en:debounceCrank = 0;
      du:debounceCrank++;
    note: // Wait X amout of time to make sure we have a stead temperature reading.
    state INACTIVE
      en:dout_GlowPlug = INACTIVE;
      en:count_GlowPlug = 0;
      state CRANKING
      state RESET_CRANK
        en:state_CrankTimerExpired = INACTIVE;
      (default) -> CRANKING
      CRANKING -> RESET_CRANK : [(state_Engine != ENGINE_CRANKING)...
          | && (CD_GLOW_PLUG_CRANKING_FUNCTION != NON_FUNCTIONAL)]
    state AFTERGLOW
      en:dout_GlowPlug = ACTIVE;
      du:debounceCount--;
      ex:glowPlugAfterGlowDone = ACTIVE;
      en:count_GlowPlug = 0;
    state PreheatWaitTime(temp) [function]
      (default) -> (junction 1709) : /* Glow plug preheat time */
      (junction 1709) -> (junction 1710) [order 1] : [temp < CD_GLOW_PLUG_TEMP_LEVEL1]
      (junction 1709) -> (junction 1711) [order 2]
      (junction 1710) -> (junction 1718) : {debounceCount = CD_GLOW_PLUG_WAIT_TIME_LEVEL0;}
      (junction 1711) -> (junction 1712) [order 1] : [temp < CD_GLOW_PLUG_TEMP_LEVEL2]
      (junction 1711) -> (junction 1713) [order 2]
      (junction 1712) -> (junction 1717) : {debounceCount = CD_GLOW_PLUG_WAIT_TIME_LEVEL1;}
      (junction 1713) -> (junction 1714) [order 1] : [temp < CD_GLOW_PLUG_TEMP_LEVEL3]
      (junction 1713) -> (junction 1715) [order 2] : {debounceCount = CD_GLOW_PLUG_WAIT_TIME_LEVEL3;}
      (junction 1714) -> (junction 1716) : {debounceCount = CD_GLOW_PLUG_WAIT_TIME_LEVEL2;}
      (junction 1716) -> (junction 1715)
      (junction 1717) -> (junction 1716)
      (junction 1718) -> (junction 1717)
      (junction 1715) -> (junction 1719)
    state PreheatTime(temp) [function]
      (default) -> (junction 1735) : /* Glow plug preheat time */
      (junction 1735) -> (junction 1736) [order 1] : [temp < CD_GLOW_PLUG_TEMP_LEVEL1]
      (junction 1735) -> (junction 1737) [order 2]
      (junction 1736) -> (junction 1744) : {debounceCount = CD_GLOW_PLUG_PREHEAT_TIME_LEVEL0;}
      (junction 1737) -> (junction 1738) [order 1] : [temp < CD_GLOW_PLUG_TEMP_LEVEL2]
      (junction 1737) -> (junction 1739) [order 2]
      (junction 1738) -> (junction 1743) : {debounceCount = CD_GLOW_PLUG_PREHEAT_TIME_LEVEL1;}
      (junction 1739) -> (junction 1740) [order 1] : [temp < CD_GLOW_PLUG_TEMP_LEVEL3]
      (junction 1739) -> (junction 1741) [order 2] : {debounceCount = CD_GLOW_PLUG_PREHEAT_TIME_LEVEL3;}
      (junction 1740) -> (junction 1742) : {debounceCount = CD_GLOW_PLUG_PREHEAT_TIME_LEVEL2;}
      (junction 1742) -> (junction 1741)
      (junction 1743) -> (junction 1742)
      (junction 1744) -> (junction 1743)
      (junction 1741) -> (junction 1745)
    state AfterglowTime(temp) [function]
      (default) -> (junction 1761) : /* Glow plug preheat time */
      (junction 1761) -> (junction 1762) [order 1] : [temp < CD_GLOW_PLUG_TEMP_LEVEL1]
      (junction 1761) -> (junction 1763) [order 2]
      (junction 1762) -> (junction 1770) : {debounceCount = CD_GLOW_PLUG_AFTERGLOW_TIME_LEVEL0;}
      (junction 1763) -> (junction 1764) [order 1] : [temp < CD_GLOW_PLUG_TEMP_LEVEL2]
      (junction 1763) -> (junction 1765) [order 2]
      (junction 1764) -> (junction 1769) : {debounceCount = CD_GLOW_PLUG_AFTERGLOW_TIME_LEVEL1;}
      (junction 1765) -> (junction 1766) [order 1] : [temp < CD_GLOW_PLUG_TEMP_LEVEL3]
      (junction 1765) -> (junction 1767) [order 2] : {debounceCount = CD_GLOW_PLUG_AFTERGLOW_TIME_LEVEL3;}
      (junction 1766) -> (junction 1768) : {debounceCount = CD_GLOW_PLUG_AFTERGLOW_TIME_LEVEL2;}
      (junction 1768) -> (junction 1767)
      (junction 1769) -> (junction 1768)
      (junction 1770) -> (junction 1769)
      (junction 1767) -> (junction 1771)
    state PREHEAT
      state PREGLOW_WAIT
        en:dout_GlowPlug = INACTIVE;
        en:cycleCount++;
        du:debounceCount--;
      state PREGLOW
        du:debounceCount--;
        du:count_GlowPlug = debounceCount;
        note: We only want the output active if the count is greater than zero.  If it equals zero we want to have
            | the output off.  That way when we enter the loop and the counter is set to zero the glow plugs
            | will not flash on for 1 count.
        (default) -> (junction 1789)
        (junction 1789) -> (junction 1790) [order 1] : [(debounceCount != 0)]
        (junction 1790) -> (junction 1792) : {
            | dout_GlowPlug = ACTIVE;
            | }
        (junction 1789) -> (junction 1791) [order 2] : {
            | dout_GlowPlug = INACTIVE;
            | }
        (junction 1792) -> (junction 1791)
      (default) -> PREGLOW
      PREGLOW -> PREGLOW_WAIT : [(debounceCount == 0)]
          | {
          | PreheatWaitTime(MachineTemp);
          | }
      PREGLOW_WAIT -> PREGLOW : [(debounceCount == 0)]
          | {
          | PreheatTime(MachineTemp);
          | }
    INACTIVE -> GLOW_DURING_CRANK [order 2] : [(CD_GLOW_PLUG_CRANKING_FUNCTION != NON_FUNCTIONAL)...
        | && (state_VBattery != OORL)...
        | && (state_Engine == ENGINE_CRANKING)...
        | && ((state_GlowPlugOutput == NORMAL) || (state_GlowPlugOutput == FAILURE))...
        | && (state_GlowPlugRelay == NORMAL)...
        | && (state_CrankTimerExpired != ACTIVE)]
    GLOW_DURING_CRANK -> INACTIVE : [(state_Engine != ENGINE_CRANKING)...
        | || !((state_GlowPlugOutput == NORMAL) || (state_GlowPlugOutput == FAILURE))...
        | || (state_GlowPlugRelay != NORMAL)...
        | || (state_VBattery == OORL)...
        | || (state_System != SYS_NORMAL)...
        | || ((debounceCrank >= CD_GLOW_PLUG_DEBOUNCE_CRANK)...
        |    && (CD_GLOW_PLUG_DEBOUNCE_CRANK != UNLIMITED))]
    (default) -> WAIT_STARTUP
    WAIT_STARTUP -> INACTIVE : [(debounceCrank > 3)]
    INACTIVE -> AFTERGLOW [order 1] : [(CD_GLOW_PLUG_AFTERGLOW_FUNCTION != NON_FUNCTIONAL)...
        | && (state_Engine == ENGINE_RUNNING)...
        | && ((state_GlowPlugOutput == NORMAL) || (state_GlowPlugOutput == FAILURE))...
        | && (state_GlowPlugRelay == NORMAL)...
        | && (glowPlugAfterGlowDone == INACTIVE)]
        | {
        | AfterglowTime(MachineTemp);
        | }
    AFTERGLOW -> INACTIVE : [(debounceCount == 0)...
        | || !((state_GlowPlugOutput == NORMAL) || (state_GlowPlugOutput == FAILURE))...
        | || (state_GlowPlugRelay != NORMAL)...
        | || (state_System != SYS_NORMAL)]
    PREHEAT -> INACTIVE : [(state_Engine == ENGINE_RUNNING)...
        | || (state_Engine == ENGINE_CRANKING)...
        | || !((state_GlowPlugOutput == NORMAL) || (state_GlowPlugOutput == FAILURE))...
        | || (state_GlowPlugRelay != NORMAL)...
        | || (state_System != SYS_NORMAL)...
        | || (state_VBattery == OORL)...
        | || (cycleCount >= CD_GLOW_PLUG_PREHEAT_COUNTER)]
        | {
        | cycleCount = CD_GLOW_PLUG_PREHEAT_COUNTER;
        | }
    INACTIVE -> PREHEAT [order 3] : [(CD_GLOW_PLUG_PREHEAT_FUNCTION != NON_FUNCTIONAL)...
        | && (cycleCount < CD_GLOW_PLUG_PREHEAT_COUNTER)...
        | && (state_VBattery != OORL)...
        | && ((CD_GLOW_PLUG_OFF_WITH_LOW_BATTERY == NON_FUNCTIONAL) || (state_VBattery == NORMAL) || (state_VBattery == HIGH))...
        | && ((state_GlowPlugOutput == NORMAL) || (state_GlowPlugOutput == FAILURE))...
        | && (state_GlowPlugRelay == NORMAL)...
        | && (state_Engine == ENGINE_NOT_RUNNING)...
        | && (state_System == SYS_NORMAL)]
        | {
        | PreheatTime(MachineTemp);
        | }
  (default) -> NON_FUNCTIONAL
  NON_FUNCTIONAL -> ECU [order 1] : [CD_ECU_FUNCTION == ECU_J1939...
      | && CD_GLOW_PLUG_ECU_COUNTDOWN_FUNCTION != NON_FUNCTIONAL]
  NON_FUNCTIONAL -> NORMAL [order 2]
  data: temp function_input uint16; temp function_input uint16; temp function_input uint16; state_Engine input uint8; state_VBattery input uint8; state_System input uint8; MachineTemp input uint16; time_GlowPlug input uint16; ecu_GlowPlug input uint8; state_ecuECT input uint8; dout_GlowPlug output boolean; state_GlowPlugOutput input uint8; state_GlowPlugRelay input uint8; CD_GLOW_PLUG_PREHEAT_FUNCTION parameter uint8; CD_GLOW_PLUG_TEMP_LEVEL1 parameter uint16; CD_GLOW_PLUG_PREHEAT_TIME_LEVEL0 parameter uint16; CD_GLOW_PLUG_TEMP_LEVEL2 parameter uint16; CD_GLOW_PLUG_TEMP_LEVEL3 parameter uint16; CD_GLOW_PLUG_WAIT_TIME_LEVEL1 parameter uint16; CD_GLOW_PLUG_PREHEAT_TIME_LEVEL2 parameter uint16; CD_GLOW_PLUG_PREHEAT_TIME_LEVEL3 parameter uint16; CD_GLOW_PLUG_WAIT_TIME_LEVEL2 parameter uint16; CD_GLOW_PLUG_WAIT_TIME_LEVEL3 parameter uint16; CD_GLOW_PLUG_WAIT_TIME_LEVEL0 parameter uint16; CD_GLOW_PLUG_PREHEAT_TIME_LEVEL1 parameter uint16; CD_GLOW_PLUG_AFTERGLOW_FUNCTION parameter uint8; CD_GLOW_PLUG_AFTERGLOW_TIME_LEVEL0 parameter uint16; CD_GLOW_PLUG_AFTERGLOW_TIME_LEVEL1 parameter uint16; CD_GLOW_PLUG_AFTERGLOW_TIME_LEVEL2 parameter uint16; CD_GLOW_PLUG_AFTERGLOW_TIME_LEVEL3 parameter uint16; CD_GLOW_PLUG_CRANKING_FUNCTION parameter uint8; CD_GLOW_PLUG_OFF_WITH_LOW_BATTERY parameter uint8; debounceCount local uint16 =0; cycleCount local uint16 =0; glowPlugAfterGlowDone local boolean =0; CD_GLOW_PLUG_PREHEAT_COUNTER parameter uint8; count_GlowPlug output uint16; ENGINE_CRANKING parameter uint8; ENGINE_NOT_RUNNING parameter uint8; ENGINE_RUNNING parameter uint8; NORMAL parameter uint8; ACTIVE parameter uint8; INACTIVE parameter uint8; SYS_NORMAL parameter uint8; HIGH parameter uint8; state_CrankTimerExpired local uint8; debounceCrank local uint16 =0; CD_GLOW_PLUG_DEBOUNCE_CRANK parameter uint16; UNLIMITED parameter uint16; NON_FUNCTIONAL parameter uint8; OORL parameter uint8; FAILURE parameter uint8; CD_GLOW_PLUG_ECU_COUNTDOWN_FUNCTION parameter uint8; CD_ECU_FUNCTION parameter uint8; ECU_J1939 parameter uint8
  data cycleCount settings: description=Counts the number of cycles the glow plugs are active.
  data count_GlowPlug settings: description=Used to output counter to panel.

== stateflow chart MX_Gtwy_Control/Inputs/Throttle/Throttle_Pos ==
  state THROTTLE_PRIMARY [parallel]
    settings: executionOrder=1
    state NON_FUNCTIONAL
      en:state_ThrottlePrimary = NORMAL;
      en:pos_ThrottlePrimary = 0;
    state OORL
      state DEBOUNCE
        en:debouncePrimary = 0;
        du:debouncePrimary++;
      state NORMAL
        en:state_ThrottlePrimary = OORL;
        en:pos_ThrottlePrimary = 0;
      (default) -> THROTTLE_PRIMARY.OORL.DEBOUNCE
      THROTTLE_PRIMARY.OORL.DEBOUNCE -> THROTTLE_PRIMARY.OORL.NORMAL : [(debouncePrimary >= CD_THROTTLE_PRIMARY_DEBOUNCE_OORL)...
          | || (state_ThrottlePrimary == OORL)]
    state NORMAL
      en:state_ThrottlePrimary = NORMAL;
      en:pos_ThrottlePrimary = pos_ThrottlePrimary_In;
      du:pos_ThrottlePrimary = pos_ThrottlePrimary_In;
    state OORH
      state NORMAL
        en:state_ThrottlePrimary = OORH;
        en:pos_ThrottlePrimary = 0;
      state DEBOUNCE
        en:debouncePrimary = 0;
        du:debouncePrimary++;
      (default) -> THROTTLE_PRIMARY.OORH.DEBOUNCE
      THROTTLE_PRIMARY.OORH.DEBOUNCE -> THROTTLE_PRIMARY.OORH.NORMAL : [(debouncePrimary >= CD_THROTTLE_PRIMARY_DEBOUNCE_OORH)...
          | || (state_ThrottlePrimary == OORH)]
    state DEBOUNCE_NORMAL
      state DEBOUNCE
        en:debouncePrimary = 0;
        du:debouncePrimary++;
      state NORMAL
        en:state_ThrottlePrimary = NORMAL;
      (default) -> THROTTLE_PRIMARY.DEBOUNCE_NORMAL.DEBOUNCE
      THROTTLE_PRIMARY.DEBOUNCE_NORMAL.DEBOUNCE -> THROTTLE_PRIMARY.DEBOUNCE_NORMAL.NORMAL : [((CD_THROTTLE_PRIMARY_DEBOUNCE_NORMAL != UNLIMITED)...
          | && (debouncePrimary >= CD_THROTTLE_PRIMARY_DEBOUNCE_NORMAL))]
    (default) -> NON_FUNCTIONAL
    NON_FUNCTIONAL -> THROTTLE_PRIMARY.NORMAL : [(CD_THROTTLE_PRIMARY_FUNCTION != NON_FUNCTIONAL)]
    THROTTLE_PRIMARY.NORMAL -> OORL [order 2] : [(rm_ThrottlePrimary <= CD_THROTTLE_PRIMARY_OORL)...
        | && (state_SensorSupply2 == NORMAL)]
    THROTTLE_PRIMARY.NORMAL -> OORH [order 1] : [(rm_ThrottlePrimary >= CD_THROTTLE_PRIMARY_OORH)...
        | && (state_SensorSupply2 == NORMAL)]
    DEBOUNCE_NORMAL -> OORL [order 3] : [(rm_ThrottlePrimary <= CD_THROTTLE_PRIMARY_OORL)]
    OORL -> DEBOUNCE_NORMAL : [(rm_ThrottlePrimary > CD_THROTTLE_PRIMARY_OORL)...
        | || (state_SensorSupply2 != NORMAL)]
    DEBOUNCE_NORMAL -> OORH [order 2] : [(rm_ThrottlePrimary >= CD_THROTTLE_PRIMARY_OORH)]
    OORH -> DEBOUNCE_NORMAL : [(rm_ThrottlePrimary < CD_THROTTLE_PRIMARY_OORH)...
        | || (state_SensorSupply2 != NORMAL)]
    DEBOUNCE_NORMAL -> THROTTLE_PRIMARY.NORMAL [order 1] : [(state_ThrottlePrimary == NORMAL)]
  state THROTTLE_POSITION [parallel]
    settings: executionOrder=2
    state NORMAL
      state VALID
        du:percent_Throttle = pos_ThrottlePrimary;
        en:derate_Throttle = 100; %No Derate
      state NO_THROTTLE
        en:percent_Throttle = CD_THROTTLE_FAULT_DERATE * 10;  // Value is percent (0 to 1000), mulitply by 10 to get correct resolution
        en:derate_Throttle = CD_THROTTLE_FAULT_DERATE;
      (default) -> VALID
      VALID -> NO_THROTTLE : [(state_ThrottlePrimary != NORMAL)...
          | || (state_SensorSupply2 != NORMAL)]
      NO_THROTTLE -> VALID : [(state_ThrottlePrimary == NORMAL)...
          | && (state_SensorSupply2 == NORMAL)]
    state PARKED_REGEN
      en: percent_Throttle = 0;
    (default) -> THROTTLE_POSITION.NORMAL
    THROTTLE_POSITION.NORMAL -> PARKED_REGEN : [(din_DPFKey == ACTIVE)]
    PARKED_REGEN -> THROTTLE_POSITION.NORMAL : [(din_DPFKey == INACTIVE)]
  data: pos_ThrottlePrimary_In input uint16; rm_ThrottlePrimary input uint16; state_ThrottlePrimary output uint8; debouncePrimary local uint16 =0; OORH parameter uint8; OORL parameter uint8; NON_FUNCTIONAL parameter uint8; NORMAL parameter uint8; CD_THROTTLE_PRIMARY_FUNCTION parameter uint8; CD_THROTTLE_PRIMARY_OORL parameter uint16; CD_THROTTLE_PRIMARY_OORH parameter uint16; CD_THROTTLE_PRIMARY_DEBOUNCE_OORL parameter uint16; pos_ThrottlePrimary output uint16; state_SensorSupply2 input uint8; CD_THROTTLE_PRIMARY_DEBOUNCE_OORH parameter uint16; CD_THROTTLE_PRIMARY_DEBOUNCE_NORMAL parameter uint16; UNLIMITED parameter uint16; derate_Throttle output uint8; percent_Throttle output uint16; CD_THROTTLE_FAULT_DERATE parameter uint8; din_DPFKey input boolean; ACTIVE parameter uint8; INACTIVE parameter uint8

== stateflow chart MX_Gtwy_Control/Outputs/PB_Valve/PBValve ==
  settings: executeAtInitialization=1
  state INACTIVE
    en:
    dout_PBValve = INACTIVE;
  state ACTIVE
    en:
    dout_PBValve = ACTIVE;
  (default) -> INACTIVE
  INACTIVE -> ACTIVE : [(CD_PBVALVE_FUNCTION != NON_FUNCTIONAL )...
      | && (((aux_Flow == AUX_FLOW_HIGH) && (state_AuxFlow == ACTIVE)) || (din_TravelPressureSwitch == ACTIVE))...
      | && (state_PBValveOutput == NORMAL)...
      | && (state_PBValveRelay == NORMAL)]
  ACTIVE -> INACTIVE : [(  ((aux_Flow != AUX_FLOW_HIGH) || (state_AuxFlow == INACTIVE)) && (din_TravelPressureSwitch == INACTIVE))...
      | || (state_PBValveOutput != NORMAL)...
      | || (state_PBValveRelay != NORMAL)]
  data: dout_PBValve output boolean; ACTIVE parameter uint8; NORMAL parameter uint8; INACTIVE parameter uint8; CD_PBVALVE_FUNCTION parameter uint8; aux_Flow input uint8; din_TravelPressureSwitch input boolean; NON_FUNCTIONAL parameter uint8; state_PBValveOutput input uint8; state_PBValveRelay input uint8; state_AuxFlow input uint8; AUX_FLOW_HIGH parameter uint8

== stateflow chart MX_Gtwy_Control/Error_Codes_Array/Throttle Cal State ==
  state Wait
    en:
    state_ThrottleCal = NORMAL;
  state Ready
    en:
    state_ThrottleCal = ee_ThrottleCalState;
    du:
    state_ThrottleCal = ee_ThrottleCalState;
  (default) -> Wait
  Wait -> Ready : [flag_InitEERead &&...
      | CD_CAN_THROTTLE_FUNCTION ~= NON_FUNCTIONAL]
  data: flag_InitEERead input boolean; state_ThrottleCal output uint8; NORMAL parameter uint8; ee_ThrottleCalState data_store_memory double; CD_CAN_THROTTLE_FUNCTION parameter uint8; NON_FUNCTIONAL parameter uint8
